simulink model "curtain_brake_encoder_dc_step"  (R2020b)
settings: Solver=ode23t  StartTime=0.0  StopTime=inf  RelTol=1e-3
model workspace (MATLAB code in the model file):
  | r_r=50e-3; %Radius of roller
  | a=5; % Height of curtain
  | b=4; % Width of curtain
  | c=2e-3; % Thickness of
  | g=9.8;
  | m_r=10; %mass of inner roller
  | m_w = 25/2*b; % mass of counter weights
  | f_c=1; %N/(m/s)/m friction of hanging curtain
  | f_r=0.1; %Nm/(rad/s) friction of roller
  | rho=1300 %kg/(m3) density of PVC
  | Td_B=16; %Derivative time constant for barrier damping
  | K_B = 400; % Gain for barrier
  | N_B = 1e3; % Max deriv gain for barrier
  | F_b = 400; %Nm max friction break torque
  | w_b = 1e-3; %Rad/sec when max friction torque reached
  | f_b = F_b/w_b; % Slope for linear part of friction model
  | V_b = 10; % Threshold voltage for releasing break
libraries: fl_lib x7, nesl_utility x5, simulink x2, pid_lib x1, simulink_extras x1, ee_lib x1, eeQuadratureDecoder x1
settings Model: EnablePacing=on  Creator=kjartan  LastModifiedBy=kjartan
settings DataIOCC: ReturnWorkspaceOutputs=off
settings DebuggingCC: IgnoredZcDiagnostic=none  ArithmeticOperatorsInVariantConditions=warning
settings SFSimCC: CustomCodeUndefinedFunction=UseInterfaceOnly

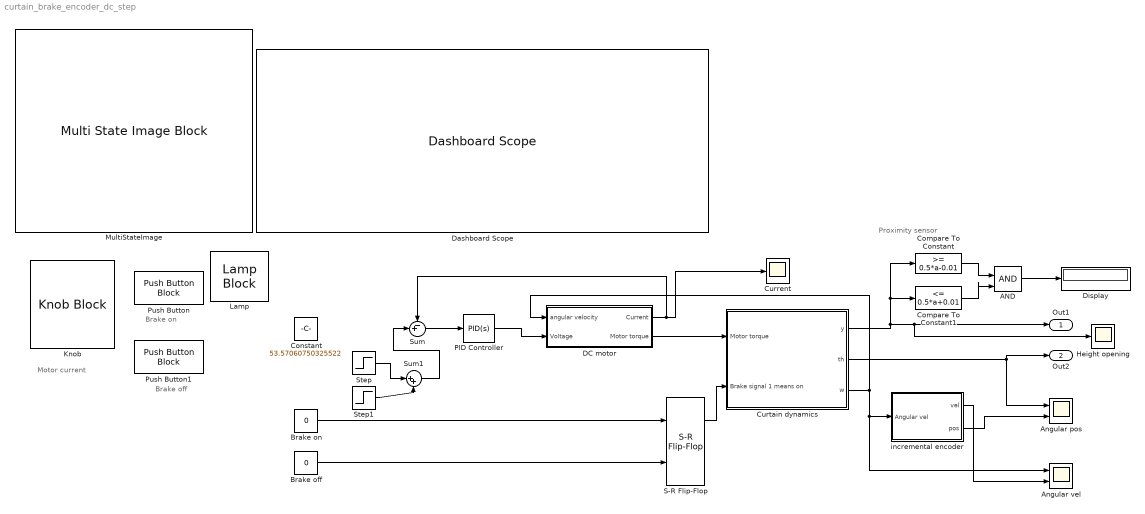
== system curtain_brake_encoder_dc_step ==
  note: Proximity sensor
  note: Motor current
  note: Brake
      | off
  note: Brake
      | on
  AND : Logic  IconShape=distinctive  AllPortsSameDT=off  OutDataTypeStr=boolean
  Angular pos : Scope  NumInputPorts=2
  Angular vel : Scope  NumInputPorts=2
  Brake off : Constant  Value=0  OutDataTypeStr=boolean
  Brake on : Constant  Value=0  OutDataTypeStr=boolean
  Compare To Constant : simulink/Logic and Bit Operations/Compare To Constant  relop=>=  const=0.5*a-0.01  OutDataTypeStr=uint8
  Compare To Constant1 : simulink/Logic and Bit Operations/Compare To Constant  relop=<=  const=0.5*a+0.01  OutDataTypeStr=boolean
  Constant : Constant  Value=53.57060750325522
  Current : Scope
  Curtain dynamics : Subsystem
  DC motor : Subsystem
  Dashboard Scope : DashboardScope  Ymin=-0.5  Ymax=6
  Display : Display
  Height opening : Scope
  Knob : KnobBlock  ScaleMin=-100
  Lamp : LampBlock
  MultiStateImage : MultiStateImageBlock
  PID Controller : pid_lib/PID Controller  Controller=PID  Form=Ideal  TimeDomain=Continuous-time  UseExternalTs=off  SampleTime=-1  IntegratorMethod=Forward Euler  FilterMethod=Forward Euler  ControllerParametersSource=internal  P=10  I=1/0.04  D=0  UseFilter=on  N=100  TunerSelectOption=Transfer Function Based (PID Tuner App)  InitialConditionSource=internal  InitialConditionForIntegrator=0  InitialConditionForFilter=0  DifferentiatorICPrevScaledInput=0  ExternalReset=none  TrackingMode=off  Kt=1  LimitOutput=off  SatLimitsSource=internal  UpperSaturationLimit=inf  LowerSaturationLimit=-inf  LinearizeAsGain=on  AntiWindupMode=none  Kb=1  POutMin=[]  POutMax=[]  IOutMin=[]  IOutMax=[]  DOutMin=[]  DOutMax=[]  NOutMin=[]  NOutMax=[]  SumOutMin=[]  SumOutMax=[]  SaturationOutMin=[]  SaturationOutMax=[]  PParamMin=[]  PParamMax=[]  IParamMin=[]  IParamMax=[]  DParamMin=[]  DParamMax=[]  NParamMin=[]  NParamMax=[]  KbParamMin=[]  KbParamMax=[]  KtParamMin=[]  KtParamMax=[]  KbOutMin=[]  KbOutMax=[]  KtOutMin=[]  KtOutMax=[]  IntegratorOutMin=[]  IntegratorOutMax=[]  FilterOutMin=[]  FilterOutMax=[]  SumI1OutMin=[]  SumI1OutMax=[]  SumI2OutMin=[]  SumI2OutMax=[]  SumI3OutMin=[]  SumI3OutMax=[]  SumI4OutMin=[]  SumI4OutMax=[]  SumDOutMin=[]  SumDOutMax=[]  DifferentiatorOutMin=[]  DifferentiatorOutMax=[]  FilterDiffOutCoefMin=[]  FilterDiffOutCoefMax=[]  ReciprocalOutMin=[]  ReciprocalOutMax=[]  SumDenOutMin=[]  SumDenOutMax=[]  SumNumOutMin=[]  SumNumOutMax=[]  DivideOutMin=[]  DivideOutMax=[]  UdiffTsProdOutMin=[]  UdiffTsProdOutMax=[]  NTsProdOutMin=[]  NTsProdOutMax=[]  UintegralTsProdOutMin=[]  UintegralTsProdOutMax=[]  UngainTsProdOutMin=[]  UngainTsProdOutMax=[]  IntegratorContinuousStateAttributes=''  IntegratorStateMustResolveToSignalObject=off  IntegratorRTWStateStorageClass=Auto  FilterContinuousStateAttributes=''  FilterStateMustResolveToSignalObject=off  FilterRTWStateStorageClass=Auto  ParallelPVariant=Passthrough  IdealPVariant=InternalParameters  IVariant=InternalParameters  DVariant=InternalParameters  IntegratorVariant=Continuous  SatVariant=Disabled  AWVariant=Passthrough  PCopyVariant=Disabled  TRVariant=Disabled  FdbkBlocksVariant=Forward  IdealPFdbkVariant=Disabled  SatFdbkVariant=Disabled  DerivativeFilterVariant=ContFilter  NVariant=InternalParameters  NCopyVariant=Disabled  FilterICVariant=InternalICsFilter  IntegratorICVariant=InternalICs  ExternalResetVariant=Disabled  TRSumVariant=Passthrough  SumFdbkVariant=Disabled  SumVariant=Sum_PID  TsampFilterVariant=InternalTs  TsampNgainVariant=Passthrough  TsampIntegralVariant=Passthrough  ExternalSaturationOutDataTypeStr=Inherit: Same as second input
  Push Button : PushButtonBlock
  Push Button1 : PushButtonBlock
  S-R Flip-Flop : simulink_extras/Flip Flops/S-R Flip-Flop  initial_condition=0
  Step : Step  Time=0  Before=53  After=53+50  SampleTime=0
  Step1 : Step  Time=0.2  After=-50  SampleTime=0
  Sum : Sum  Inputs=-+|
  Sum1 : Sum  Inputs=|++
  incremental encoder : Subsystem
  Out1 : Outport  VectorParamsAs1DForOutWhenUnconnected=off
  Out2 : Outport  Port=2  VectorParamsAs1DForOutWhenUnconnected=off
  wires:
  Curtain dynamics/1(y) -> Compare To Constant1/1, Compare To Constant/1, Out1/1, Height opening/1
  Curtain dynamics/2(th) -> Out2/1, Angular pos/1
  S-R Flip-Flop/1 -> Curtain dynamics/2("Brake signal 1 means on ")
  Curtain dynamics/3(w) -> DC motor/1(angular velocity), Angular vel/1, incremental encoder/1(Angular vel)
  incremental encoder/2(pos) -> Angular pos/2
  incremental encoder/1(vel) -> Angular vel/2
  Compare To Constant/1 -> AND/1
  Compare To Constant1/1 -> AND/2
  AND/1 -> Display/1
  Brake off/1 -> S-R Flip-Flop/2
  Brake on/1 -> S-R Flip-Flop/1
  DC motor/2(Motor torque) -> Curtain dynamics/1(Motor torque)
  DC motor/1(Current) -> Sum/1, Current/1
  Sum/1 -> PID Controller/1
  PID Controller/1 -> DC motor/2(Voltage)
  Step1/1 -> Sum1/2
  Step/1 -> Sum1/1
  Sum1/1 -> Sum/2

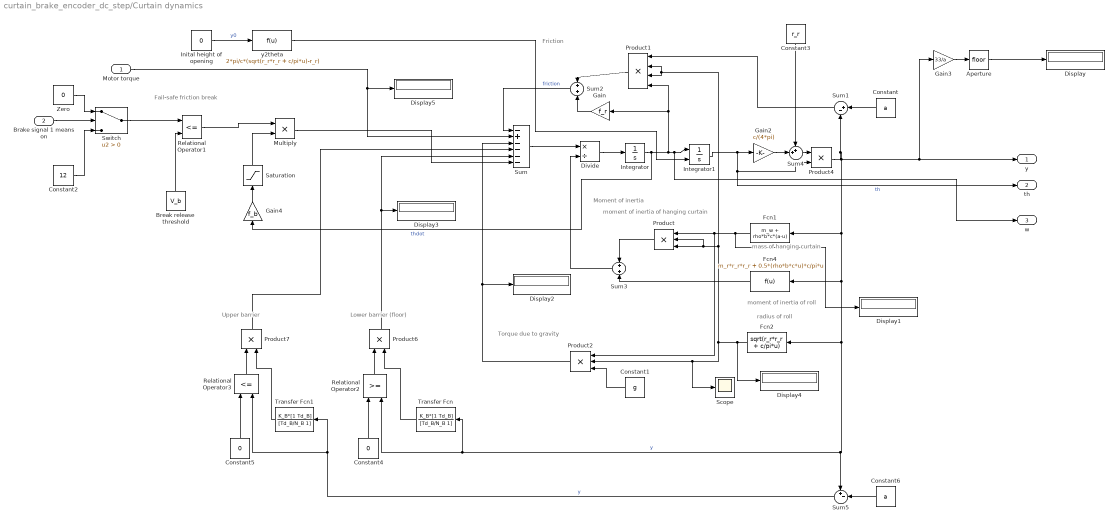
== system curtain_brake_encoder_dc_step/Curtain dynamics ==
  note: Fail-safe friction break
  note: Torque due to gravity
  note: Moment of inertia
  note: Friction
  note: Lower barrier (floor)
  note: moment of inertia of hanging curtain
  note: moment of inertia of roll
  note: radius of roll
  note: mass of hanging curtain
  note: Upper barrier
  Motor torque : Inport
  "Brake signal 1 means on " : Inport  Port=2
  Aperture : Rounding
  Break release threshold : Constant  Value=V_b
  Constant : Constant  Value=a
  Constant1 : Constant  Value=g
  Constant2 : Constant  Value=12
  Constant3 : Constant  Value=r_r
  Constant4 : Constant  Value=0
  Constant5 : Constant  Value=0
  Constant6 : Constant  Value=a
  Display{,1..5} (6 alike) : Display
  Divide : Product  Inputs=*/
  Fcn1 : Fcn  Expr=m_w + rho*b*c*(a-u)
  Fcn2 : Fcn  Expr=sqrt(r_r*r_r + c/pi*u)
  Fcn4 : Fcn  Expr=m_r*r_r*r_r + 0.5*(rho*b*c*u)*c/pi*u
  Gain : Gain  Gain=f_r
  Gain2 : Gain  Gain=c/(4*pi)
  Gain3 : Gain  Gain=33/a
  Gain4 : Gain  Gain=f_b
  Inital height of opening : Constant  Value=0
  Integrator : Integrator
  Integrator1 : Integrator  InitialConditionSource=external  InitialCondition=12*2*pi
  Multiply : Product
  Product : Product  Inputs=3
  Product1 : Product  Inputs=4
  Product2 : Product  Inputs=3
  Product4 : Product
  Product6 : Product
  Product7 : Product
  Relational Operator1 : RelationalOperator  Operator=<=  OutDataTypeStr=boolean  RndMeth=Simplest
  Relational Operator2 : RelationalOperator  OutDataTypeStr=boolean  RndMeth=Simplest
  Relational Operator3 : RelationalOperator  Operator=<=  OutDataTypeStr=boolean  RndMeth=Simplest
  Saturation : Saturate  UpperLimit=F_b  LowerLimit=-F_b
  Scope : Scope
  Sum : Sum  Inputs=-+----
  Sum1 : Sum  Inputs=|+-
  Sum2 : Sum  Inputs=+|+
  Sum3 : Sum  Inputs=+|+
  Sum4 : Sum  Inputs=++|
  Sum5 : Sum  Inputs=+-|
  Switch : Switch  Criteria=u2 > Threshold
  Transfer Fcn : TransferFcn  Numerator=K_B*[1 Td_B]  Denominator=[Td_B/N_B 1]
  Transfer Fcn1 : TransferFcn  Numerator=K_B*[1 Td_B]  Denominator=[Td_B/N_B 1]
  Zero : Constant  Value=0  OutDataTypeStr=Inherit: Inherit via back propagation
  y2theta : Fcn  Expr=2*pi/c*(sqrt(r_r*r_r + c/pi*u)-r_r)
  y : Outport  VectorParamsAs1DForOutWhenUnconnected=off
  th : Outport  Port=2  VectorParamsAs1DForOutWhenUnconnected=off
  w : Outport  Port=3
  wires:
  Fcn4/1 -> Sum3/2
  Constant/1 -> Sum1/1
  Gain/1 -> Sum2/2
  Constant1/1 -> Product2/3
  Relational Operator3/1 -> Product7/1
  Divide/1 -> Integrator/1
  Sum4/1 -> Product4/1
  Constant3/1 -> Sum4/1
  Constant4/1 -> Relational Operator2/1
  Constant6/1 -> Sum5/2
  Sum5/1 -> Transfer Fcn1/1, Relational Operator3/2  "y"
  Fcn1/1 -> Display1/1, Product2/1, Product/1
  Product4/1 -> Gain3/1, y/1, Sum5/1, Transfer Fcn/1, Relational Operator2/2, Fcn2/1, Fcn4/1, Fcn1/1, Sum1/2  "y"
  Integrator1/1 -> th/1, Product4/2, Gain2/1  "th"
  Sum2/1 -> Sum/1  "friction"
  Fcn2/1 -> Display4/1, Product1/2, Product1/3, Product/2, Product/3, Scope/1, Product2/2
  Product1/1 -> Sum2/1
  Product/1 -> Sum3/1
  Sum3/1 -> Divide/2
  Relational Operator2/1 -> Product6/1
  Integrator/1 -> Gain4/1, Product1/4, Gain/1, w/1, Integrator1/1  "thdot"
  Product7/1 -> Sum/4
  Constant5/1 -> Relational Operator3/1
  Sum1/1 -> Product1/1
  Product6/1 -> Display3/1, Sum/5
  Product2/1 -> Display2/1, Sum/3
  Transfer Fcn/1 -> Product6/2
  Sum/1 -> Divide/1
  Gain2/1 -> Sum4/2
  Transfer Fcn1/1 -> Product7/2
  Gain3/1 -> Aperture/1
  Aperture/1 -> Display/1
  Inital height of opening/1 -> y2theta/1  "y0"
  y2theta/1 -> Integrator1/2
  "Brake signal 1 means on "/1 -> Switch/2
  Break release threshold/1 -> Relational Operator1/2
  Gain4/1 -> Saturation/1
  Saturation/1 -> Multiply/2
  Multiply/1 -> Sum/6
  Motor torque/1 -> Sum/2, Display5/1
  Relational Operator1/1 -> Multiply/1
  Zero/1 -> Switch/1
  Constant2/1 -> Switch/3
  Switch/1 -> Relational Operator1/1

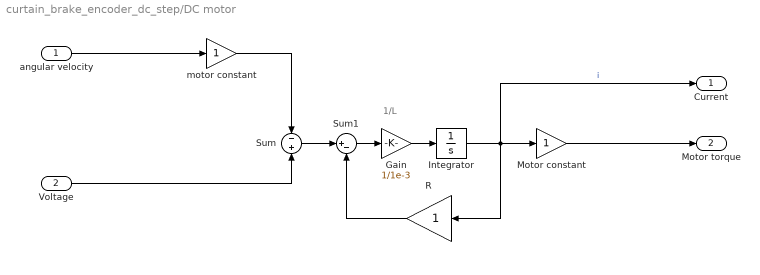
== system curtain_brake_encoder_dc_step/DC motor ==
  note: 1/L
  angular velocity : Inport
  Voltage : Inport  Port=2
  Gain : Gain  Gain=1/1e-3
  Integrator : Integrator
  Motor constant : Gain
  R : Gain
  Sum : Sum  Inputs=-+
  Sum1 : Sum  Inputs=|+-
  motor constant : Gain
  Current : Outport
  Motor torque : Outport  Port=2
  wires:
  motor constant/1 -> Sum/1
  Voltage/1 -> Sum/2
  Integrator/1 -> Current/1, R/1, Motor constant/1  "i"
  Sum1/1 -> Gain/1
  Sum/1 -> Sum1/1
  R/1 -> Sum1/2
  Gain/1 -> Integrator/1
  angular velocity/1 -> motor constant/1
  Motor constant/1 -> Motor torque/1

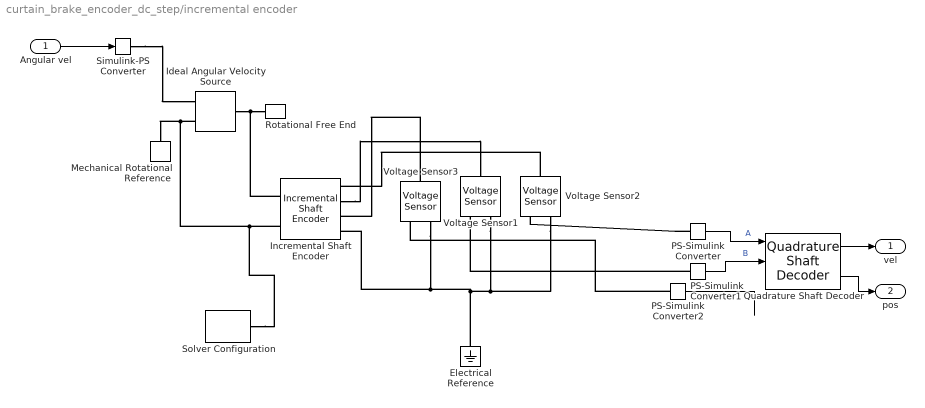
== system curtain_brake_encoder_dc_step/incremental encoder ==
  Angular vel : Inport
  Electrical Reference : fl_lib/Electrical/Electrical Elements/Electrical Reference
  Ideal Angular Velocity Source : fl_lib/Mechanical/Mechanical Sources/Ideal Angular Velocity Source
  Incremental Shaft Encoder : ee_lib/Sensors & Transducers/Incremental Shaft Encoder  NPulse=36  Vm=5 V  angle0=0 deg
      settings: SourceProductBaseCode=PS  InternalSimscapePortConfiguration="{\"Bottom\":[],\"Left\":[{\"id\":\"R\",\"label\":\"R\",\"type\":\"foundation.mechanical.rotational.rotational\"},{\"id\":\"C\",\"label\":\"C\",\"type\":\"foundation.mechanical.rotational.rotational\"}],\"Right\":[{\"id\":\"A\",\"label\":\"A\",\"type\":\"foundation.electrical.electrical\"},{\"id\":\"B\",\"label\":\"B\",\"type\":\"foundation.electrical.electrical\"},{\"id\":\"Z\",\"label\":\"Z\",\"type\":\"foundation.electrical.electrical\"},{\"id\":\"GND\",\"label\":\"REF\",\"type\":\"foundation.electrical.electrical\"}],\"Top\":[]}"
  Mechanical Rotational Reference : fl_lib/Mechanical/Rotational Elements/Mechanical Rotational Reference
  PS-Simulink Converter : nesl_utility/PS-Simulink Converter  PhysicalDomain=network_engine_domain  SubClassName=ps_output  LeftPortType=input  RightPortType=output  PseudoPeriodic=off  Frequencies=[]  Unit=inherit  AffineConversion=off
  PS-Simulink Converter1 : nesl_utility/PS-Simulink Converter  PhysicalDomain=network_engine_domain  SubClassName=ps_output  LeftPortType=input  RightPortType=output  PseudoPeriodic=off  Frequencies=[]  Unit=inherit  AffineConversion=off
  PS-Simulink Converter2 : nesl_utility/PS-Simulink Converter  PhysicalDomain=network_engine_domain  SubClassName=ps_output  LeftPortType=input  RightPortType=output  PseudoPeriodic=off  Frequencies=[]  Unit=inherit  AffineConversion=off
  Quadrature Shaft Decoder : eeQuadratureDecoder/Quadrature Shaft Decoder  NPulse=36  Ts=1e-4
      settings: SourceProductBaseCode=PS
  Rotational Free End : fl_lib/Mechanical/Rotational Elements/Rotational Free End  w_specify=off  w_priority=None  w=0 rad/s  w_nominal_specify=off  w_nominal_unit=rad/s  w_nominal_value=1
      settings: SourceProductBaseCode=SS
  Simulink-PS Converter : nesl_utility/Simulink-PS Converter  PhysicalDomain=network_engine_domain  SubClassName=ps_input  LeftPortType=input  RightPortType=output  PseudoPeriodic=off  Frequencies=[]  NoiseDistribution=none  NoiseParameters=[]  Unit=1  AffineConversion=off  FilteringAndDerivatives=provide  SimscapeFilterOrder=1  InputFilterTimeConstant=0.001  UdotUserProvided=0
  Solver Configuration : nesl_utility/Solver Configuration  PhysicalDomain=network_engine_domain  SubClassName=solver  LeftPortType=input  RightPortType=generic  Tones=[]  Harmonics=[]  SimulateNoise=off  Profile=off  UseLocalSampling=off  EnableSwitchedLinearOptims=on  FrequencyDomain=off  UseCCode=off  RelTol=0.001  AbsTol=1e-06  MinStep=1e-09  ToWorkspace=off  RevertToSquareIcSolve=off  NumHomotopyIterations=100  PhasorMode=off  PhasorModeNumHarmonics=1  DoDC=off  ResidualTolerance=1e-09  UseLocalSolver=off  LocalSolverChoice=NE_BACKWARD_EULER_ADVANCER  LocalSolverSampleTime=0.001  DoFixedCost=off  MaxNonlinIter=3  MaxModeIter=2  ComputeImpulses=off  ImpulseIterations=2  LinearAlgebra=auto  EquationFormulation=NE_TIME_EF  DelaysMemoryBudget=1024  AutomaticFiltering=on  FilteringTimeConstant=0.001  PartitionStorageMethod=AS_NEEDED  PartitionMemoryBudget=1024  PartitionMethod=ROBUST
  Voltage Sensor1 : fl_lib/Electrical/Electrical Sensors/Voltage Sensor
  Voltage Sensor2 : fl_lib/Electrical/Electrical Sensors/Voltage Sensor
  Voltage Sensor3 : fl_lib/Electrical/Electrical Sensors/Voltage Sensor
  vel : Outport
  pos : Outport  Port=2
  wires:
  PS-Simulink Converter1/1 -> Quadrature Shaft Decoder/2  "B"
  PS-Simulink Converter/1 -> Quadrature Shaft Decoder/1  "A"
  Angular vel/1 -> Simulink-PS Converter/1
  Quadrature Shaft Decoder/1 -> vel/1
  Quadrature Shaft Decoder/2 -> pos/1
  PS-Simulink Converter2/1 -> ?
  connections:
  Voltage Sensor2/RConn2 -- Electrical Reference/LConn1 -- Incremental Shaft Encoder/RConn4 -- Voltage Sensor3/RConn2 -- Voltage Sensor1/RConn2
  Incremental Shaft Encoder/LConn1 -- Ideal Angular Velocity Source/LConn1 -- Rotational Free End/LConn1
  Incremental Shaft Encoder/LConn2 -- Solver Configuration/RConn1 -- Ideal Angular Velocity Source/RConn2 -- Mechanical Rotational Reference/LConn1
  Incremental Shaft Encoder/RConn2 -- Voltage Sensor1/LConn1
  Voltage Sensor2/LConn1 -- Incremental Shaft Encoder/RConn1
  PS-Simulink Converter1/LConn1 -- Voltage Sensor1/RConn1
  Simulink-PS Converter/RConn1 -- Ideal Angular Velocity Source/RConn1
  PS-Simulink Converter/LConn1 -- Voltage Sensor2/RConn1
  Voltage Sensor3/LConn1 -- Incremental Shaft Encoder/RConn3
  PS-Simulink Converter2/LConn1 -- Voltage Sensor3/RConn1
